simulink model "fft_2p_4kc_18i_18o_core"  (R2021a)
settings: Solver=VariableStepDiscrete  StartTime=0.0  StopTime=2^11*3  RelTol=1e-3
undefined symbols (not defined in the file or in its folder): i
libraries: xbsIndex_r4 x48628, casper_library_misc x100, casper_library_delays x10
(part 163 of 338)

== system fft_2p_4kc_18i_18o_core/fft/fft_biplex/biplex_core/fft_stage_5/butterfly_direct/bus_expand ==
  bus_in : Inport
  reinterpret1 : xbsIndex_r4/Reinterpret  force_arith_type=on  arith_type=Unsigned  force_bin_pt=on  bin_pt=0  block_type=reinterpret
  reinterpret2 : xbsIndex_r4/Reinterpret  force_arith_type=on  arith_type=Unsigned  force_bin_pt=on  bin_pt=0  block_type=reinterpret
  slice1 : xbsIndex_r4/Slice  nbits=576  boolean_output=off  mode=Upper Bit Location + Width  bit1=-576  base1=MSB of Input  bit0=0  base0=LSB of Input  dbl_ovrd=off  block_type=slice
  slice2 : xbsIndex_r4/Slice  nbits=576  boolean_output=off  mode=Upper Bit Location + Width  bit1=0  base1=MSB of Input  bit0=0  base0=LSB of Input  dbl_ovrd=off  block_type=slice
  msb_out2 : Outport  VectorParamsAs1DForOutWhenUnconnected=off
  lsb_out1 : Outport  Port=2  VectorParamsAs1DForOutWhenUnconnected=off
  wires:
  bus_in/1 -> slice2/1, slice1/1
  slice2/1 -> reinterpret2/1
  reinterpret2/1 -> msb_out2/1
  slice1/1 -> reinterpret1/1
  reinterpret1/1 -> lsb_out1/1

== system fft_2p_4kc_18i_18o_core/fft/fft_biplex/biplex_core/fft_stage_5/butterfly_direct/bus_norm0 ==
  din : Inport
  bussify : Subsystem  AncestorBlock=casper_library_flow_control/bus_create  mask(bus_create){inputNum=64}
      mask prompts: inputNum "Number of inputs:"
      mask init:
      | bus_create_init(gcb,...
      |     'inputNum', inputNum);
  conv{1..64} (64 alike) : xbsIndex_r4/Convert  gui_display_data_type=Fixed-point  arith_type="Signed  (2's comp)"  n_bits=21  bin_pt=18  float_type=Single  exp_bits=8  fraction_bits=24  quantization=Truncate  overflow=Wrap  en=off  latency=0  pipeline=on  dbl_ovrd=off  block_type=convert
  debus : Subsystem  AncestorBlock=casper_library_flow_control/bus_expand  mask(bus_expand){mode=divisions of arbitrary size, outputNum=2, outputWidth=[20 20 20 20 20 20 20 20 20 20 20 20 20 20 20 20 20 20 20 20 20 20 20 20 20 20 20 20 20 20 20 20 20 20 20 20 20 20 20 20 20 20 20 20 20 20 20 20 20 20 20 20 20 20 20 20 20 20 20 20 20 20 20 20], outputBinaryPt=[17 17 17 17 17 17 17 17 17 17 17 17 17 17 17 17 17 17 17 17 17 17 17 17 17 17 17 17 17 17 17 17 17 17 17 17 17 17 17 17 17 17 17 17 17 17 17 17 17 17 17 17 17 17 17 17 17 17 17 17 17 17 17 17], outputArithmeticType=[1 1 1 1 1 1 1 1 1 1 1 1 1 1 1 1 1 1 1 1 1 1 1 1 1 1 1 1 1 1 1 1 1 1 1 1 1 1 1 1 1 1 1 1 1 1 1 1 1 1 1 1 1 1 1 1 1 1 1 1 1 1 1 1], show_format=on, outputToWorkspace=off, variablePrefix=, outputToModelAsWell=on}
      mask prompts: outputNum "Number of outputs:"; outputWidth "Output width:"; outputBinaryPt "Output binary point position:"; outputArithmeticType "Output arithmetic type (ufix=0, fix=1, bool=2):"; show_format "Print format string?"; outputToWorkspace "Output to workspace?"; variablePrefix "Variable name prefix:"; outputToModelAsWell "Output to model as well?"
      mask help:
      | Expands a 'bus' made using a bus_create or Xilinx
      | concat block.
      | The input will be divided into the specified number
      | of outputs with the specified arithmetic types.
      | Divisions are made from most significant bit.
      mask init: same as fft_2p_4kc_18i_18o_core/bus_expand
      settings: AttributesFormatString=64 outputs: f20.17,f20.17,f20.17,f20.17,f20.17,f20.17,f20.17,f20.17,f20.17,f20.17,f20.17,f20.17,f20.17,f20.17,f20.17,f20.17,f20.17,f20.17,f20.17,f20.17,f20.17,f20.17,f20.17,f20.17,f20.17,f20.17,f20.17,f20.17,f20.17,f20.17,f20.17,f20.17,f20.17,f20.17,f20.17,f20.17,f20.17,f20.17,f20.17,f20.17,f20.17,f20.17,f20.17,f20.17,f20.17,f20.17,f20.17,f20.17,f20.17,f20.17,f20.17,f20.17,f20.17,f20.17,f20.17,f20.17,f20.17,f20.17,f20.17,f20.17,f20.17,f20.17,f20.17,f20.17,
      mask TypeOptions mode: divisions of equal size | divisions of arbitrary size
      mask Callback mode: %bus_expand_callback();
      mask Callback outputWidth: %bus_expand_callback();
      mask Callback outputToWorkspace: %bus_expand_callback();
  dout : Outport
  wires:
  din/1 -> debus/1(bus_in)
  debus/1(msb_out64) -> conv1/1
  debus/2(out63) -> conv2/1
  debus/3(out62) -> conv3/1
  debus/4(out61) -> conv4/1
  debus/5(out60) -> conv5/1
  debus/6(out59) -> conv6/1
  debus/7(out58) -> conv7/1
  debus/8(out57) -> conv8/1
  debus/9(out56) -> conv9/1
  debus/10(out55) -> conv10/1
  debus/11(out54) -> conv11/1
  debus/12(out53) -> conv12/1
  debus/13(out52) -> conv13/1
  debus/14(out51) -> conv14/1
  debus/15(out50) -> conv15/1
  debus/16(out49) -> conv16/1
  debus/17(out48) -> conv17/1
  debus/18(out47) -> conv18/1
  debus/19(out46) -> conv19/1
  debus/20(out45) -> conv20/1
  debus/21(out44) -> conv21/1
  debus/22(out43) -> conv22/1
  debus/23(out42) -> conv23/1
  debus/24(out41) -> conv24/1
  debus/25(out40) -> conv25/1
  debus/26(out39) -> conv26/1
  debus/27(out38) -> conv27/1
  debus/28(out37) -> conv28/1
  debus/29(out36) -> conv29/1
  debus/30(out35) -> conv30/1
  debus/31(out34) -> conv31/1
  debus/32(out33) -> conv32/1
  debus/33(out32) -> conv33/1
  debus/34(out31) -> conv34/1
  debus/35(out30) -> conv35/1
  debus/36(out29) -> conv36/1
  debus/37(out28) -> conv37/1
  debus/38(out27) -> conv38/1
  debus/39(out26) -> conv39/1
  debus/40(out25) -> conv40/1
  debus/41(out24) -> conv41/1
  debus/42(out23) -> conv42/1
  debus/43(out22) -> conv43/1
  debus/44(out21) -> conv44/1
  debus/45(out20) -> conv45/1
  debus/46(out19) -> conv46/1
  debus/47(out18) -> conv47/1
  debus/48(out17) -> conv48/1
  debus/49(out16) -> conv49/1
  debus/50(out15) -> conv50/1
  debus/51(out14) -> conv51/1
  debus/52(out13) -> conv52/1
  debus/53(out12) -> conv53/1
  debus/54(out11) -> conv54/1
  debus/55(out10) -> conv55/1
  debus/56(out9) -> conv56/1
  debus/57(out8) -> conv57/1
  debus/58(out7) -> conv58/1
  debus/59(out6) -> conv59/1
  debus/60(out5) -> conv60/1
  debus/61(out4) -> conv61/1
  debus/62(out3) -> conv62/1
  debus/63(out2) -> conv63/1
  debus/64(lsb_out1) -> conv64/1
  conv1/1 -> bussify/1(in1)
  conv2/1 -> bussify/2(in2)
  conv3/1 -> bussify/3(in3)
  conv4/1 -> bussify/4(in4)
  conv5/1 -> bussify/5(in5)
  conv6/1 -> bussify/6(in6)
  conv7/1 -> bussify/7(in7)
  conv8/1 -> bussify/8(in8)
  conv9/1 -> bussify/9(in9)
  conv10/1 -> bussify/10(in10)
  conv11/1 -> bussify/11(in11)
  conv12/1 -> bussify/12(in12)
  conv13/1 -> bussify/13(in13)
  conv14/1 -> bussify/14(in14)
  conv15/1 -> bussify/15(in15)
  conv16/1 -> bussify/16(in16)
  conv17/1 -> bussify/17(in17)
  conv18/1 -> bussify/18(in18)
  conv19/1 -> bussify/19(in19)
  conv20/1 -> bussify/20(in20)
  conv21/1 -> bussify/21(in21)
  conv22/1 -> bussify/22(in22)
  conv23/1 -> bussify/23(in23)
  conv24/1 -> bussify/24(in24)
  conv25/1 -> bussify/25(in25)
  conv26/1 -> bussify/26(in26)
  conv27/1 -> bussify/27(in27)
  conv28/1 -> bussify/28(in28)
  conv29/1 -> bussify/29(in29)
  conv30/1 -> bussify/30(in30)
  conv31/1 -> bussify/31(in31)
  conv32/1 -> bussify/32(in32)
  conv33/1 -> bussify/33(in33)
  conv34/1 -> bussify/34(in34)
  conv35/1 -> bussify/35(in35)
  conv36/1 -> bussify/36(in36)
  conv37/1 -> bussify/37(in37)
  conv38/1 -> bussify/38(in38)
  conv39/1 -> bussify/39(in39)
  conv40/1 -> bussify/40(in40)
  conv41/1 -> bussify/41(in41)
  conv42/1 -> bussify/42(in42)
  conv43/1 -> bussify/43(in43)
  conv44/1 -> bussify/44(in44)
  conv45/1 -> bussify/45(in45)
  conv46/1 -> bussify/46(in46)
  conv47/1 -> bussify/47(in47)
  conv48/1 -> bussify/48(in48)
  conv49/1 -> bussify/49(in49)
  conv50/1 -> bussify/50(in50)
  conv51/1 -> bussify/51(in51)
  conv52/1 -> bussify/52(in52)
  conv53/1 -> bussify/53(in53)
  conv54/1 -> bussify/54(in54)
  conv55/1 -> bussify/55(in55)
  conv56/1 -> bussify/56(in56)
  conv57/1 -> bussify/57(in57)
  conv58/1 -> bussify/58(in58)
  conv59/1 -> bussify/59(in59)
  conv60/1 -> bussify/60(in60)
  conv61/1 -> bussify/61(in61)
  conv62/1 -> bussify/62(in62)
  conv63/1 -> bussify/63(in63)
  conv64/1 -> bussify/64(in64)
  bussify/1(bus_out) -> dout/1

== system fft_2p_4kc_18i_18o_core/fft/fft_biplex/biplex_core/fft_stage_5/butterfly_direct/bus_norm1 ==
  din : Inport
  bussify : Subsystem  AncestorBlock=casper_library_flow_control/bus_create  mask(bus_create){inputNum=64}
      mask prompts: inputNum "Number of inputs:"
      mask init:
      | bus_create_init(gcb,...
      |     'inputNum', inputNum);
  conv{1..64} (64 alike) : xbsIndex_r4/Convert  gui_display_data_type=Fixed-point  arith_type="Signed  (2's comp)"  n_bits=21  bin_pt=18  float_type=Single  exp_bits=8  fraction_bits=24  quantization=Truncate  overflow=Wrap  en=off  latency=0  pipeline=on  dbl_ovrd=off  block_type=convert
  debus : Subsystem  AncestorBlock=casper_library_flow_control/bus_expand  mask(bus_expand){mode=divisions of arbitrary size, outputNum=2, outputWidth=[20 20 20 20 20 20 20 20 20 20 20 20 20 20 20 20 20 20 20 20 20 20 20 20 20 20 20 20 20 20 20 20 20 20 20 20 20 20 20 20 20 20 20 20 20 20 20 20 20 20 20 20 20 20 20 20 20 20 20 20 20 20 20 20], outputBinaryPt=[18 18 18 18 18 18 18 18 18 18 18 18 18 18 18 18 18 18 18 18 18 18 18 18 18 18 18 18 18 18 18 18 18 18 18 18 18 18 18 18 18 18 18 18 18 18 18 18 18 18 18 18 18 18 18 18 18 18 18 18 18 18 18 18], outputArithmeticType=[1 1 1 1 1 1 1 1 1 1 1 1 1 1 1 1 1 1 1 1 1 1 1 1 1 1 1 1 1 1 1 1 1 1 1 1 1 1 1 1 1 1 1 1 1 1 1 1 1 1 1 1 1 1 1 1 1 1 1 1 1 1 1 1], show_format=on, outputToWorkspace=off, variablePrefix=, outputToModelAsWell=on}
      mask prompts: outputNum "Number of outputs:"; outputWidth "Output width:"; outputBinaryPt "Output binary point position:"; outputArithmeticType "Output arithmetic type (ufix=0, fix=1, bool=2):"; show_format "Print format string?"; outputToWorkspace "Output to workspace?"; variablePrefix "Variable name prefix:"; outputToModelAsWell "Output to model as well?"
      mask help:
      | Expands a 'bus' made using a bus_create or Xilinx
      | concat block.
      | The input will be divided into the specified number
      | of outputs with the specified arithmetic types.
      | Divisions are made from most significant bit.
      mask init: same as fft_2p_4kc_18i_18o_core/bus_expand
      settings: AttributesFormatString=64 outputs: f20.18,f20.18,f20.18,f20.18,f20.18,f20.18,f20.18,f20.18,f20.18,f20.18,f20.18,f20.18,f20.18,f20.18,f20.18,f20.18,f20.18,f20.18,f20.18,f20.18,f20.18,f20.18,f20.18,f20.18,f20.18,f20.18,f20.18,f20.18,f20.18,f20.18,f20.18,f20.18,f20.18,f20.18,f20.18,f20.18,f20.18,f20.18,f20.18,f20.18,f20.18,f20.18,f20.18,f20.18,f20.18,f20.18,f20.18,f20.18,f20.18,f20.18,f20.18,f20.18,f20.18,f20.18,f20.18,f20.18,f20.18,f20.18,f20.18,f20.18,f20.18,f20.18,f20.18,f20.18,
      mask TypeOptions mode: divisions of equal size | divisions of arbitrary size
      mask Callback mode: %bus_expand_callback();
      mask Callback outputWidth: %bus_expand_callback();
      mask Callback outputToWorkspace: %bus_expand_callback();
  dout : Outport
  wires:
  din/1 -> debus/1(bus_in)
  debus/1(msb_out64) -> conv1/1
  debus/2(out63) -> conv2/1
  debus/3(out62) -> conv3/1
  debus/4(out61) -> conv4/1
  debus/5(out60) -> conv5/1
  debus/6(out59) -> conv6/1
  debus/7(out58) -> conv7/1
  debus/8(out57) -> conv8/1
  debus/9(out56) -> conv9/1
  debus/10(out55) -> conv10/1
  debus/11(out54) -> conv11/1
  debus/12(out53) -> conv12/1
  debus/13(out52) -> conv13/1
  debus/14(out51) -> conv14/1
  debus/15(out50) -> conv15/1
  debus/16(out49) -> conv16/1
  debus/17(out48) -> conv17/1
  debus/18(out47) -> conv18/1
  debus/19(out46) -> conv19/1
  debus/20(out45) -> conv20/1
  debus/21(out44) -> conv21/1
  debus/22(out43) -> conv22/1
  debus/23(out42) -> conv23/1
  debus/24(out41) -> conv24/1
  debus/25(out40) -> conv25/1
  debus/26(out39) -> conv26/1
  debus/27(out38) -> conv27/1
  debus/28(out37) -> conv28/1
  debus/29(out36) -> conv29/1
  debus/30(out35) -> conv30/1
  debus/31(out34) -> conv31/1
  debus/32(out33) -> conv32/1
  debus/33(out32) -> conv33/1
  debus/34(out31) -> conv34/1
  debus/35(out30) -> conv35/1
  debus/36(out29) -> conv36/1
  debus/37(out28) -> conv37/1
  debus/38(out27) -> conv38/1
  debus/39(out26) -> conv39/1
  debus/40(out25) -> conv40/1
  debus/41(out24) -> conv41/1
  debus/42(out23) -> conv42/1
  debus/43(out22) -> conv43/1
  debus/44(out21) -> conv44/1
  debus/45(out20) -> conv45/1
  debus/46(out19) -> conv46/1
  debus/47(out18) -> conv47/1
  debus/48(out17) -> conv48/1
  debus/49(out16) -> conv49/1
  debus/50(out15) -> conv50/1
  debus/51(out14) -> conv51/1
  debus/52(out13) -> conv52/1
  debus/53(out12) -> conv53/1
  debus/54(out11) -> conv54/1
  debus/55(out10) -> conv55/1
  debus/56(out9) -> conv56/1
  debus/57(out8) -> conv57/1
  debus/58(out7) -> conv58/1
  debus/59(out6) -> conv59/1
  debus/60(out5) -> conv60/1
  debus/61(out4) -> conv61/1
  debus/62(out3) -> conv62/1
  debus/63(out2) -> conv63/1
  debus/64(lsb_out1) -> conv64/1
  conv1/1 -> bussify/1(in1)
  conv2/1 -> bussify/2(in2)
  conv3/1 -> bussify/3(in3)
  conv4/1 -> bussify/4(in4)
  conv5/1 -> bussify/5(in5)
  conv6/1 -> bussify/6(in6)
  conv7/1 -> bussify/7(in7)
  conv8/1 -> bussify/8(in8)
  conv9/1 -> bussify/9(in9)
  conv10/1 -> bussify/10(in10)
  conv11/1 -> bussify/11(in11)
  conv12/1 -> bussify/12(in12)
  conv13/1 -> bussify/13(in13)
  conv14/1 -> bussify/14(in14)
  conv15/1 -> bussify/15(in15)
  conv16/1 -> bussify/16(in16)
  conv17/1 -> bussify/17(in17)
  conv18/1 -> bussify/18(in18)
  conv19/1 -> bussify/19(in19)
  conv20/1 -> bussify/20(in20)
  conv21/1 -> bussify/21(in21)
  conv22/1 -> bussify/22(in22)
  conv23/1 -> bussify/23(in23)
  conv24/1 -> bussify/24(in24)
  conv25/1 -> bussify/25(in25)
  conv26/1 -> bussify/26(in26)
  conv27/1 -> bussify/27(in27)
  conv28/1 -> bussify/28(in28)
  conv29/1 -> bussify/29(in29)
  conv30/1 -> bussify/30(in30)
  conv31/1 -> bussify/31(in31)
  conv32/1 -> bussify/32(in32)
  conv33/1 -> bussify/33(in33)
  conv34/1 -> bussify/34(in34)
  conv35/1 -> bussify/35(in35)
  conv36/1 -> bussify/36(in36)
  conv37/1 -> bussify/37(in37)
  conv38/1 -> bussify/38(in38)
  conv39/1 -> bussify/39(in39)
  conv40/1 -> bussify/40(in40)
  conv41/1 -> bussify/41(in41)
  conv42/1 -> bussify/42(in42)
  conv43/1 -> bussify/43(in43)
  conv44/1 -> bussify/44(in44)
  conv45/1 -> bussify/45(in45)
  conv46/1 -> bussify/46(in46)
  conv47/1 -> bussify/47(in47)
  conv48/1 -> bussify/48(in48)
  conv49/1 -> bussify/49(in49)
  conv50/1 -> bussify/50(in50)
  conv51/1 -> bussify/51(in51)
  conv52/1 -> bussify/52(in52)
  conv53/1 -> bussify/53(in53)
  conv54/1 -> bussify/54(in54)
  conv55/1 -> bussify/55(in55)
  conv56/1 -> bussify/56(in56)
  conv57/1 -> bussify/57(in57)
  conv58/1 -> bussify/58(in58)
  conv59/1 -> bussify/59(in59)
  conv60/1 -> bussify/60(in60)
  conv61/1 -> bussify/61(in61)
  conv62/1 -> bussify/62(in62)
  conv63/1 -> bussify/63(in63)
  conv64/1 -> bussify/64(in64)
  bussify/1(bus_out) -> dout/1

== system fft_2p_4kc_18i_18o_core/fft/fft_biplex/biplex_core/fft_stage_5/butterfly_direct/bus_relational ==
  a : Inport
  b : Inport  Port=2
  a_debus : Subsystem  AncestorBlock=casper_library_flow_control/bus_expand  mask(bus_expand){mode=divisions of arbitrary size, outputNum=2, outputWidth=[4], outputBinaryPt=[0], outputArithmeticType=[0], show_format=on, outputToWorkspace=off, variablePrefix=, outputToModelAsWell=on}
      mask prompts: outputNum "Number of outputs:"; outputWidth "Output width:"; outputBinaryPt "Output binary point position:"; outputArithmeticType "Output arithmetic type (ufix=0, fix=1, bool=2):"; show_format "Print format string?"; outputToWorkspace "Output to workspace?"; variablePrefix "Variable name prefix:"; outputToModelAsWell "Output to model as well?"
      mask help:
      | Expands a 'bus' made using a bus_create or Xilinx
      | concat block.
      | The input will be divided into the specified number
      | of outputs with the specified arithmetic types.
      | Divisions are made from most significant bit.
      mask init: same as fft_2p_4kc_18i_18o_core/bus_expand
      settings: AttributesFormatString=1 outputs: uf4.0,
      mask TypeOptions mode: divisions of equal size | divisions of arbitrary size
      mask Callback mode: %bus_expand_callback();
      mask Callback outputWidth: %bus_expand_callback();
      mask Callback outputToWorkspace: %bus_expand_callback();
  b_debus : Subsystem  AncestorBlock=casper_library_flow_control/bus_expand  mask(bus_expand){mode=divisions of arbitrary size, outputNum=2, outputWidth=[4  4  4  4  4  4  4  4  4  4  4  4  4  4  4  4], outputBinaryPt=[0  0  0  0  0  0  0  0  0  0  0  0  0  0  0  0], outputArithmeticType=[0  0  0  0  0  0  0  0  0  0  0  0  0  0  0  0], show_format=on, outputToWorkspace=off, variablePrefix=, outputToModelAsWell=on}
      mask prompts: outputNum "Number of outputs:"; outputWidth "Output width:"; outputBinaryPt "Output binary point position:"; outputArithmeticType "Output arithmetic type (ufix=0, fix=1, bool=2):"; show_format "Print format string?"; outputToWorkspace "Output to workspace?"; variablePrefix "Variable name prefix:"; outputToModelAsWell "Output to model as well?"
      mask help:
      | Expands a 'bus' made using a bus_create or Xilinx
      | concat block.
      | The input will be divided into the specified number
      | of outputs with the specified arithmetic types.
      | Divisions are made from most significant bit.
      mask init: same as fft_2p_4kc_18i_18o_core/bus_expand
      settings: AttributesFormatString=16 outputs: uf4.0,uf4.0,uf4.0,uf4.0,uf4.0,uf4.0,uf4.0,uf4.0,uf4.0,uf4.0,uf4.0,uf4.0,uf4.0,uf4.0,uf4.0,uf4.0,
      mask TypeOptions mode: divisions of equal size | divisions of arbitrary size
      mask Callback mode: %bus_expand_callback();
      mask Callback outputWidth: %bus_expand_callback();
      mask Callback outputToWorkspace: %bus_expand_callback();
  bussify : Subsystem  AncestorBlock=casper_library_flow_control/bus_create  mask(bus_create){inputNum=16}
      mask prompts: inputNum "Number of inputs:"
      mask init:
      | bus_create_init(gcb,...
      |     'inputNum', inputNum);
  relational{1..16} (16 alike) : xbsIndex_r4/Relational  mode=a!=b  op_type=Bool  en=off  latency=1  dbl_ovrd=off  block_type=relational
  a!=b : Outport
  wires:
  a/1 -> a_debus/1(bus_in)
  b/1 -> b_debus/1(bus_in)
  a_debus/1(msb_lsb_out1) -> relational1/1, relational2/1, relational3/1, relational4/1, relational5/1, relational6/1, relational7/1, relational8/1, relational9/1, relational10/1, relational11/1, relational12/1, relational13/1, relational14/1, relational15/1, relational16/1
  b_debus/1(msb_out16) -> relational1/2
  b_debus/2(out15) -> relational2/2
  b_debus/3(out14) -> relational3/2
  b_debus/4(out13) -> relational4/2
  b_debus/5(out12) -> relational5/2
  b_debus/6(out11) -> relational6/2
  b_debus/7(out10) -> relational7/2
  b_debus/8(out9) -> relational8/2
  b_debus/9(out8) -> relational9/2
  b_debus/10(out7) -> relational10/2
  b_debus/11(out6) -> relational11/2
  b_debus/12(out5) -> relational12/2
  b_debus/13(out4) -> relational13/2
  b_debus/14(out3) -> relational14/2
  b_debus/15(out2) -> relational15/2
  b_debus/16(lsb_out1) -> relational16/2
  relational1/1 -> bussify/1(in1)
  relational2/1 -> bussify/2(in2)
  relational3/1 -> bussify/3(in3)
  relational4/1 -> bussify/4(in4)
  relational5/1 -> bussify/5(in5)
  relational6/1 -> bussify/6(in6)
  relational7/1 -> bussify/7(in7)
  relational8/1 -> bussify/8(in8)
  relational9/1 -> bussify/9(in9)
  relational10/1 -> bussify/10(in10)
  relational11/1 -> bussify/11(in11)
  relational12/1 -> bussify/12(in12)
  relational13/1 -> bussify/13(in13)
  relational14/1 -> bussify/14(in14)
  relational15/1 -> bussify/15(in15)
  relational16/1 -> bussify/16(in16)
  bussify/1(bus_out) -> a!=b/1

== system fft_2p_4kc_18i_18o_core/fft/fft_biplex/biplex_core/fft_stage_5/butterfly_direct/bus_scale ==
  din : Inport
  bussify : Subsystem  AncestorBlock=casper_library_flow_control/bus_create  mask(bus_create){inputNum=64}
      mask prompts: inputNum "Number of inputs:"
      mask init:
      | bus_create_init(gcb,...
      |     'inputNum', inputNum);
  debus : Subsystem  AncestorBlock=casper_library_flow_control/bus_expand  mask(bus_expand){mode=divisions of arbitrary size, outputNum=2, outputWidth=[20 20 20 20 20 20 20 20 20 20 20 20 20 20 20 20 20 20 20 20 20 20 20 20 20 20 20 20 20 20 20 20 20 20 20 20 20 20 20 20 20 20 20 20 20 20 20 20 20 20 20 20 20 20 20 20 20 20 20 20 20 20 20 20], outputBinaryPt=[17 17 17 17 17 17 17 17 17 17 17 17 17 17 17 17 17 17 17 17 17 17 17 17 17 17 17 17 17 17 17 17 17 17 17 17 17 17 17 17 17 17 17 17 17 17 17 17 17 17 17 17 17 17 17 17 17 17 17 17 17 17 17 17], outputArithmeticType=[1 1 1 1 1 1 1 1 1 1 1 1 1 1 1 1 1 1 1 1 1 1 1 1 1 1 1 1 1 1 1 1 1 1 1 1 1 1 1 1 1 1 1 1 1 1 1 1 1 1 1 1 1 1 1 1 1 1 1 1 1 1 1 1], show_format=on, outputToWorkspace=off, variablePrefix=, outputToModelAsWell=on}
      mask prompts: outputNum "Number of outputs:"; outputWidth "Output width:"; outputBinaryPt "Output binary point position:"; outputArithmeticType "Output arithmetic type (ufix=0, fix=1, bool=2):"; show_format "Print format string?"; outputToWorkspace "Output to workspace?"; variablePrefix "Variable name prefix:"; outputToModelAsWell "Output to model as well?"
      mask help:
      | Expands a 'bus' made using a bus_create or Xilinx
      | concat block.
      | The input will be divided into the specified number
      | of outputs with the specified arithmetic types.
      | Divisions are made from most significant bit.
      mask init: same as fft_2p_4kc_18i_18o_core/bus_expand
      settings: AttributesFormatString=64 outputs: f20.17,f20.17,f20.17,f20.17,f20.17,f20.17,f20.17,f20.17,f20.17,f20.17,f20.17,f20.17,f20.17,f20.17,f20.17,f20.17,f20.17,f20.17,f20.17,f20.17,f20.17,f20.17,f20.17,f20.17,f20.17,f20.17,f20.17,f20.17,f20.17,f20.17,f20.17,f20.17,f20.17,f20.17,f20.17,f20.17,f20.17,f20.17,f20.17,f20.17,f20.17,f20.17,f20.17,f20.17,f20.17,f20.17,f20.17,f20.17,f20.17,f20.17,f20.17,f20.17,f20.17,f20.17,f20.17,f20.17,f20.17,f20.17,f20.17,f20.17,f20.17,f20.17,f20.17,f20.17,
      mask TypeOptions mode: divisions of equal size | divisions of arbitrary size
      mask Callback mode: %bus_expand_callback();
      mask Callback outputWidth: %bus_expand_callback();
      mask Callback outputToWorkspace: %bus_expand_callback();
  scale{1..64} (64 alike) : xbsIndex_r4/Scale  scale_factor=-1  dbl_ovrd=off  block_type=scale
  dout : Outport
  wires:
  din/1 -> debus/1(bus_in)
  debus/1(msb_out64) -> scale1/1
  debus/2(out63) -> scale2/1
  debus/3(out62) -> scale3/1
  debus/4(out61) -> scale4/1
  debus/5(out60) -> scale5/1
  debus/6(out59) -> scale6/1
  debus/7(out58) -> scale7/1
  debus/8(out57) -> scale8/1
  debus/9(out56) -> scale9/1
  debus/10(out55) -> scale10/1
  debus/11(out54) -> scale11/1
  debus/12(out53) -> scale12/1
  debus/13(out52) -> scale13/1
  debus/14(out51) -> scale14/1
  debus/15(out50) -> scale15/1
  debus/16(out49) -> scale16/1
  debus/17(out48) -> scale17/1
  debus/18(out47) -> scale18/1
  debus/19(out46) -> scale19/1
  debus/20(out45) -> scale20/1
  debus/21(out44) -> scale21/1
  debus/22(out43) -> scale22/1
  debus/23(out42) -> scale23/1
  debus/24(out41) -> scale24/1
  debus/25(out40) -> scale25/1
  debus/26(out39) -> scale26/1
  debus/27(out38) -> scale27/1
  debus/28(out37) -> scale28/1
  debus/29(out36) -> scale29/1
  debus/30(out35) -> scale30/1
  debus/31(out34) -> scale31/1
  debus/32(out33) -> scale32/1
  debus/33(out32) -> scale33/1
  debus/34(out31) -> scale34/1
  debus/35(out30) -> scale35/1
  debus/36(out29) -> scale36/1
  debus/37(out28) -> scale37/1
  debus/38(out27) -> scale38/1
  debus/39(out26) -> scale39/1
  debus/40(out25) -> scale40/1
  debus/41(out24) -> scale41/1
  debus/42(out23) -> scale42/1
  debus/43(out22) -> scale43/1
  debus/44(out21) -> scale44/1
  debus/45(out20) -> scale45/1
  debus/46(out19) -> scale46/1
  debus/47(out18) -> scale47/1
  debus/48(out17) -> scale48/1
  debus/49(out16) -> scale49/1
  debus/50(out15) -> scale50/1
  debus/51(out14) -> scale51/1
  debus/52(out13) -> scale52/1
  debus/53(out12) -> scale53/1
  debus/54(out11) -> scale54/1
  debus/55(out10) -> scale55/1
  debus/56(out9) -> scale56/1
  debus/57(out8) -> scale57/1
  debus/58(out7) -> scale58/1
  debus/59(out6) -> scale59/1
  debus/60(out5) -> scale60/1
  debus/61(out4) -> scale61/1
  debus/62(out3) -> scale62/1
  debus/63(out2) -> scale63/1
  debus/64(lsb_out1) -> scale64/1
  scale1/1 -> bussify/1(in1)
  scale2/1 -> bussify/2(in2)
  scale3/1 -> bussify/3(in3)
  scale4/1 -> bussify/4(in4)
  scale5/1 -> bussify/5(in5)
  scale6/1 -> bussify/6(in6)
  scale7/1 -> bussify/7(in7)
  scale8/1 -> bussify/8(in8)
  scale9/1 -> bussify/9(in9)
  scale10/1 -> bussify/10(in10)
  scale11/1 -> bussify/11(in11)
  scale12/1 -> bussify/12(in12)
  scale13/1 -> bussify/13(in13)
  scale14/1 -> bussify/14(in14)
  scale15/1 -> bussify/15(in15)
  scale16/1 -> bussify/16(in16)
  scale17/1 -> bussify/17(in17)
  scale18/1 -> bussify/18(in18)
  scale19/1 -> bussify/19(in19)
  scale20/1 -> bussify/20(in20)
  scale21/1 -> bussify/21(in21)
  scale22/1 -> bussify/22(in22)
  scale23/1 -> bussify/23(in23)
  scale24/1 -> bussify/24(in24)
  scale25/1 -> bussify/25(in25)
  scale26/1 -> bussify/26(in26)
  scale27/1 -> bussify/27(in27)
  scale28/1 -> bussify/28(in28)
  scale29/1 -> bussify/29(in29)
  scale30/1 -> bussify/30(in30)
  scale31/1 -> bussify/31(in31)
  scale32/1 -> bussify/32(in32)
  scale33/1 -> bussify/33(in33)
  scale34/1 -> bussify/34(in34)
  scale35/1 -> bussify/35(in35)
  scale36/1 -> bussify/36(in36)
  scale37/1 -> bussify/37(in37)
  scale38/1 -> bussify/38(in38)
  scale39/1 -> bussify/39(in39)
  scale40/1 -> bussify/40(in40)
  scale41/1 -> bussify/41(in41)
  scale42/1 -> bussify/42(in42)
  scale43/1 -> bussify/43(in43)
  scale44/1 -> bussify/44(in44)
  scale45/1 -> bussify/45(in45)
  scale46/1 -> bussify/46(in46)
  scale47/1 -> bussify/47(in47)
  scale48/1 -> bussify/48(in48)
  scale49/1 -> bussify/49(in49)
  scale50/1 -> bussify/50(in50)
  scale51/1 -> bussify/51(in51)
  scale52/1 -> bussify/52(in52)
  scale53/1 -> bussify/53(in53)
  scale54/1 -> bussify/54(in54)
  scale55/1 -> bussify/55(in55)
  scale56/1 -> bussify/56(in56)
  scale57/1 -> bussify/57(in57)
  scale58/1 -> bussify/58(in58)
  scale59/1 -> bussify/59(in59)
  scale60/1 -> bussify/60(in60)
  scale61/1 -> bussify/61(in61)
  scale62/1 -> bussify/62(in62)
  scale63/1 -> bussify/63(in63)
  scale64/1 -> bussify/64(in64)
  bussify/1(bus_out) -> dout/1

== system fft_2p_4kc_18i_18o_core/fft/fft_biplex/biplex_core/fft_stage_5/butterfly_direct/bus_sub ==
  a : Inport
  b : Inport  Port=2
  a_debus : Subsystem  AncestorBlock=casper_library_flow_control/bus_expand  mask(bus_expand){mode=divisions of arbitrary size, outputNum=2, outputWidth=[18  18  18  18  18  18  18  18  18  18  18  18  18  18  18  18  18  18  18  18  18  18  18  18  18  18  18  18  18  18  18  18], outputBinaryPt=[17  17  17  17  17  17  17  17  17  17  17  17  17  17  17  17  17  17  17  17  17  17  17  17  17  17  17  17  17  17  17  17], outputArithmeticType=[1  1  1  1  1  1  1  1  1  1  1  1  1  1  1  1  1  1  1  1  1  1  1  1  1  1  1  1  1  1  1  1], show_format=on, outputToWorkspace=off, variablePrefix=, outputToModelAsWell=on}
      mask prompts: outputNum "Number of outputs:"; outputWidth "Output width:"; outputBinaryPt "Output binary point position:"; outputArithmeticType "Output arithmetic type (ufix=0, fix=1, bool=2):"; show_format "Print format string?"; outputToWorkspace "Output to workspace?"; variablePrefix "Variable name prefix:"; outputToModelAsWell "Output to model as well?"
      mask help:
      | Expands a 'bus' made using a bus_create or Xilinx
      | concat block.
      | The input will be divided into the specified number
      | of outputs with the specified arithmetic types.
      | Divisions are made from most significant bit.
      mask init: same as fft_2p_4kc_18i_18o_core/bus_expand
      settings: AttributesFormatString=32 outputs: f18.17,f18.17,f18.17,f18.17,f18.17,f18.17,f18.17,f18.17,f18.17,f18.17,f18.17,f18.17,f18.17,f18.17,f18.17,f18.17,f18.17,f18.17,f18.17,f18.17,f18.17,f18.17,f18.17,f18.17,f18.17,f18.17,f18.17,f18.17,f18.17,f18.17,f18.17,f18.17,
      mask TypeOptions mode: divisions of equal size | divisions of arbitrary size
      mask Callback mode: %bus_expand_callback();
      mask Callback outputWidth: %bus_expand_callback();
      mask Callback outputToWorkspace: %bus_expand_callback();
  addsub{1..32} (32 alike) : xbsIndex_r4/AddSub  mode=Subtraction  use_carryin=off  use_carryout=off  en=off  latency=1  precision=User Defined  arith_type="Signed  (2's comp)"  n_bits=20  bin_pt=17  quantization=Truncate  overflow=Wrap  use_behavioral_HDL=on  hw_selection=Fabric  pipelined=on  dbl_ovrd=off  block_type=addsub
  b_debus : Subsystem  AncestorBlock=casper_library_flow_control/bus_expand  mask(bus_expand){mode=divisions of arbitrary size, outputNum=2, outputWidth=[19  19  19  19  19  19  19  19  19  19  19  19  19  19  19  19  19  19  19  19  19  19  19  19  19  19  19  19  19  19  19  19], outputBinaryPt=[17  17  17  17  17  17  17  17  17  17  17  17  17  17  17  17  17  17  17  17  17  17  17  17  17  17  17  17  17  17  17  17], outputArithmeticType=[1  1  1  1  1  1  1  1  1  1  1  1  1  1  1  1  1  1  1  1  1  1  1  1  1  1  1  1  1  1  1  1], show_format=on, outputToWorkspace=off, variablePrefix=, outputToModelAsWell=on}
      mask prompts: outputNum "Number of outputs:"; outputWidth "Output width:"; outputBinaryPt "Output binary point position:"; outputArithmeticType "Output arithmetic type (ufix=0, fix=1, bool=2):"; show_format "Print format string?"; outputToWorkspace "Output to workspace?"; variablePrefix "Variable name prefix:"; outputToModelAsWell "Output to model as well?"
      mask help:
      | Expands a 'bus' made using a bus_create or Xilinx
      | concat block.
      | The input will be divided into the specified number
      | of outputs with the specified arithmetic types.
      | Divisions are made from most significant bit.
      mask init: same as fft_2p_4kc_18i_18o_core/bus_expand
      settings: AttributesFormatString=32 outputs: f19.17,f19.17,f19.17,f19.17,f19.17,f19.17,f19.17,f19.17,f19.17,f19.17,f19.17,f19.17,f19.17,f19.17,f19.17,f19.17,f19.17,f19.17,f19.17,f19.17,f19.17,f19.17,f19.17,f19.17,f19.17,f19.17,f19.17,f19.17,f19.17,f19.17,f19.17,f19.17,
      mask TypeOptions mode: divisions of equal size | divisions of arbitrary size
      mask Callback mode: %bus_expand_callback();
      mask Callback outputWidth: %bus_expand_callback();
      mask Callback outputToWorkspace: %bus_expand_callback();
  op_bussify : Subsystem  AncestorBlock=casper_library_flow_control/bus_create  mask(bus_create){inputNum=32}
      mask prompts: inputNum "Number of inputs:"
      mask init:
      | bus_create_init(gcb,...
      |     'inputNum', inputNum);
  dout : Outport
  wires:
  a/1 -> a_debus/1(bus_in)
  b/1 -> b_debus/1(bus_in)
  a_debus/1(msb_out32) -> addsub1/1
  b_debus/1(msb_out32) -> addsub1/2
  a_debus/2(out31) -> addsub2/1
  b_debus/2(out31) -> addsub2/2
  a_debus/3(out30) -> addsub3/1
  b_debus/3(out30) -> addsub3/2
  a_debus/4(out29) -> addsub4/1
  b_debus/4(out29) -> addsub4/2
  a_debus/5(out28) -> addsub5/1
  b_debus/5(out28) -> addsub5/2
  a_debus/6(out27) -> addsub6/1
  b_debus/6(out27) -> addsub6/2
  a_debus/7(out26) -> addsub7/1
  b_debus/7(out26) -> addsub7/2
  a_debus/8(out25) -> addsub8/1
  b_debus/8(out25) -> addsub8/2
  a_debus/9(out24) -> addsub9/1
  b_debus/9(out24) -> addsub9/2
  a_debus/10(out23) -> addsub10/1
  b_debus/10(out23) -> addsub10/2
  a_debus/11(out22) -> addsub11/1
  b_debus/11(out22) -> addsub11/2
  a_debus/12(out21) -> addsub12/1
  b_debus/12(out21) -> addsub12/2
  a_debus/13(out20) -> addsub13/1
  b_debus/13(out20) -> addsub13/2
  a_debus/14(out19) -> addsub14/1
  b_debus/14(out19) -> addsub14/2
  a_debus/15(out18) -> addsub15/1
  b_debus/15(out18) -> addsub15/2
  a_debus/16(out17) -> addsub16/1
  b_debus/16(out17) -> addsub16/2
  a_debus/17(out16) -> addsub17/1
  b_debus/17(out16) -> addsub17/2
  a_debus/18(out15) -> addsub18/1
  b_debus/18(out15) -> addsub18/2
  a_debus/19(out14) -> addsub19/1
  b_debus/19(out14) -> addsub19/2
  a_debus/20(out13) -> addsub20/1
  b_debus/20(out13) -> addsub20/2
  a_debus/21(out12) -> addsub21/1
  b_debus/21(out12) -> addsub21/2
  a_debus/22(out11) -> addsub22/1
  b_debus/22(out11) -> addsub22/2
  a_debus/23(out10) -> addsub23/1
  b_debus/23(out10) -> addsub23/2
  a_debus/24(out9) -> addsub24/1
  b_debus/24(out9) -> addsub24/2
  a_debus/25(out8) -> addsub25/1
  b_debus/25(out8) -> addsub25/2
  a_debus/26(out7) -> addsub26/1
  b_debus/26(out7) -> addsub26/2
  a_debus/27(out6) -> addsub27/1
  b_debus/27(out6) -> addsub27/2
  a_debus/28(out5) -> addsub28/1
  b_debus/28(out5) -> addsub28/2
  a_debus/29(out4) -> addsub29/1
  b_debus/29(out4) -> addsub29/2
  a_debus/30(out3) -> addsub30/1
  b_debus/30(out3) -> addsub30/2
  a_debus/31(out2) -> addsub31/1
  b_debus/31(out2) -> addsub31/2
  a_debus/32(lsb_out1) -> addsub32/1
  b_debus/32(lsb_out1) -> addsub32/2
  addsub1/1 -> op_bussify/1(in1)
  addsub2/1 -> op_bussify/2(in2)
  addsub3/1 -> op_bussify/3(in3)
  addsub4/1 -> op_bussify/4(in4)
  addsub5/1 -> op_bussify/5(in5)
  addsub6/1 -> op_bussify/6(in6)
  addsub7/1 -> op_bussify/7(in7)
  addsub8/1 -> op_bussify/8(in8)
  addsub9/1 -> op_bussify/9(in9)
  addsub10/1 -> op_bussify/10(in10)
  addsub11/1 -> op_bussify/11(in11)
  addsub12/1 -> op_bussify/12(in12)
  addsub13/1 -> op_bussify/13(in13)
  addsub14/1 -> op_bussify/14(in14)
  addsub15/1 -> op_bussify/15(in15)
  addsub16/1 -> op_bussify/16(in16)
  addsub17/1 -> op_bussify/17(in17)
  addsub18/1 -> op_bussify/18(in18)
  addsub19/1 -> op_bussify/19(in19)
  addsub20/1 -> op_bussify/20(in20)
  addsub21/1 -> op_bussify/21(in21)
  addsub22/1 -> op_bussify/22(in22)
  addsub23/1 -> op_bussify/23(in23)
  addsub24/1 -> op_bussify/24(in24)
  addsub25/1 -> op_bussify/25(in25)
  addsub26/1 -> op_bussify/26(in26)
  addsub27/1 -> op_bussify/27(in27)
  addsub28/1 -> op_bussify/28(in28)
  addsub29/1 -> op_bussify/29(in29)
  addsub30/1 -> op_bussify/30(in30)
  addsub31/1 -> op_bussify/31(in31)
  addsub32/1 -> op_bussify/32(in32)
  op_bussify/1(bus_out) -> dout/1

== system fft_2p_4kc_18i_18o_core/fft/fft_biplex/biplex_core/fft_stage_5/butterfly_direct/munge ==
  din : Inport
  join : Subsystem  AncestorBlock=casper_library_flow_control/bus_create  mask(bus_create){inputNum=32}
      mask prompts: inputNum "Number of inputs:"
      mask init:
      | bus_create_init(gcb,...
      |     'inputNum', inputNum);
  reinterpret : xbsIndex_r4/Reinterpret  force_arith_type=on  arith_type=Unsigned  force_bin_pt=on  bin_pt=0  block_type=reinterpret
  reinterpret_out : xbsIndex_r4/Reinterpret  force_arith_type=on  arith_type=Unsigned  force_bin_pt=on  bin_pt=0  block_type=reinterpret
  split : Subsystem  AncestorBlock=casper_library_flow_control/bus_expand  mask(bus_expand){mode=divisions of arbitrary size, outputNum=32, outputWidth=[2 2 2 2 2 2 2 2 2 2 2 2 2 2 2 2 2 2 2 2 2 2 2 2 2 2 2 2 2 2 2 2], outputBinaryPt=[0 0 0 0 0 0 0 0 0 0 0 0 0 0 0 0 0 0 0 0 0 0 0 0 0 0 0 0 0 0 0 0], outputArithmeticType=[0 0 0 0 0 0 0 0 0 0 0 0 0 0 0 0 0 0 0 0 0 0 0 0 0 0 0 0 0 0 0 0], show_format=off, outputToWorkspace=off, variablePrefix=out, outputToModelAsWell=on}
      mask prompts: outputNum "Number of outputs:"; outputWidth "Output width:"; outputBinaryPt "Output binary point position:"; outputArithmeticType "Output arithmetic type (ufix=0, fix=1, bool=2):"; show_format "Print format string?"; outputToWorkspace "Output to workspace?"; variablePrefix "Variable name prefix:"; outputToModelAsWell "Output to model as well?"
      mask help:
      | Expands a 'bus' made using a bus_create or Xilinx
      | concat block.
      | The input will be divided into the specified number
      | of outputs with the specified arithmetic types.
      | Divisions are made from most significant bit.
      mask init: same as fft_2p_4kc_18i_18o_core/bus_expand
      settings: AttributesFormatString=32 outputs
      mask TypeOptions mode: divisions of equal size | divisions of arbitrary size
      mask Callback mode: %bus_expand_callback();
      mask Callback outputWidth: %bus_expand_callback();
      mask Callback outputToWorkspace: %bus_expand_callback();
  dout : Outport
  wires:
  reinterpret_out/1 -> dout/1
  din/1 -> reinterpret/1
  reinterpret/1 -> split/1(bus_in)
  join/1(bus_out) -> reinterpret_out/1
  split/1(msb_out32) -> join/1(in1)
  split/17(out16) -> join/2(in2)
  split/2(out31) -> join/3(in3)
  split/18(out15) -> join/4(in4)
  split/3(out30) -> join/5(in5)
  split/19(out14) -> join/6(in6)
  split/4(out29) -> join/7(in7)
  split/20(out13) -> join/8(in8)
  split/5(out28) -> join/9(in9)
  split/21(out12) -> join/10(in10)
  split/6(out27) -> join/11(in11)
  split/22(out11) -> join/12(in12)
  split/7(out26) -> join/13(in13)
  split/23(out10) -> join/14(in14)
  split/8(out25) -> join/15(in15)
  split/24(out9) -> join/16(in16)
  split/9(out24) -> join/17(in17)
  split/25(out8) -> join/18(in18)
  split/10(out23) -> join/19(in19)
  split/26(out7) -> join/20(in20)
  split/11(out22) -> join/21(in21)
  split/27(out6) -> join/22(in22)
  split/12(out21) -> join/23(in23)
  split/28(out5) -> join/24(in24)
  split/13(out20) -> join/25(in25)
  split/29(out4) -> join/26(in26)
  split/14(out19) -> join/27(in27)
  split/30(out3) -> join/28(in28)
  split/15(out18) -> join/29(in29)
  split/31(out2) -> join/30(in30)
  split/16(out17) -> join/31(in31)
  split/32(lsb_out1) -> join/32(in32)
